simulink model "CCMDcMotor"  (R2022b)
settings: Solver=FixedStepDiscrete  StartTime=0.0  StopTime=10.0  FixedStep=0.01  RelTol=1e-3
model workspace (MAT data in the model file):
  NvMBlockDcMtrCalData : Simulink.Signal (value not decoded)
  NvMBlockDcMtrCalDataInit : Simulink.Parameter (value not decoded)
symbols that are objects in the model workspace (value not decoded): NvMBlockDcMtrCalDataInit
undefined symbols (not defined in the file or in its folder): DTCState, NvMBlockId, P_Std_ReturnType, Tle91ChlType, Tle91CmdType
(part 2 of 3)

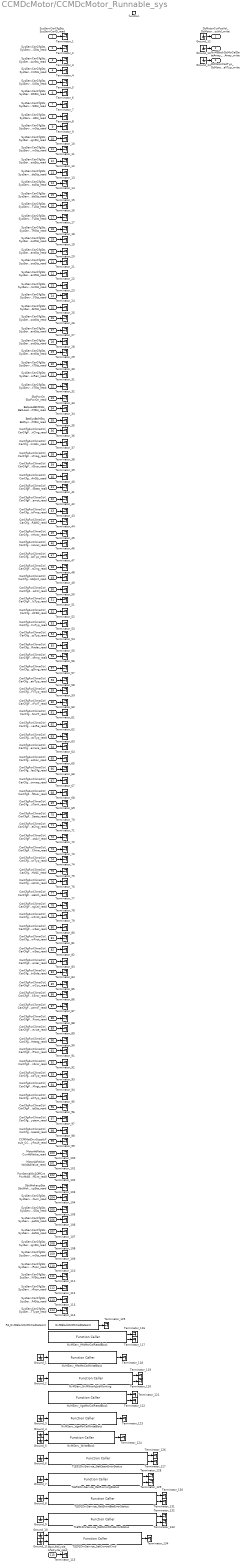
== system CCMDcMotor/CCMDcMotor_Runnable_sys ==
  SysServCarCfgSts_SysServCarID_read : Inport
  SysServCarCfgSts_SysServCCPAQSSts_read : Inport  Port=2
  SysServCarCfgSts_SysServCCPHEXSensorSts_read : Inport  Port=3
  SysServCarCfgSts_SysServCCPLin2AFUSts_read : Inport  Port=4
  SysServCarCfgSts_SysServCCPLin2AIUSts_read : Inport  Port=5
  SysServCarCfgSts_SysServCCPLin2CDSSts_read : Inport  Port=6
  SysServCarCfgSts_SysServCCPLin2PMSISts_read : Inport  Port=7
  SysServCarCfgSts_SysServCCPLin3VGASts_read : Inport  Port=8
  SysServCarCfgSts_SysServDUAirVentSnrSts_read : Inport  Port=9
  SysServCarCfgSts_SysServDUBlowerPwmSignSts_read : Inport  Port=10
  SysServCarCfgSts_SysServDUBlowerPwmSts_read : Inport  Port=11
  SysServCarCfgSts_SysServDUDCDefrostSts_read : Inport  Port=12
  SysServCarCfgSts_SysServDUDCModeSts_read : Inport  Port=13
  SysServCarCfgSts_SysServDUDCRecSts_read : Inport  Port=14
  SysServCarCfgSts_SysServDUDCSModeSts_read : Inport  Port=15
  SysServCarCfgSts_SysServDUDCSTLSts_read : Inport  Port=16
  SysServCarCfgSts_SysServDUDCTLSts_read : Inport  Port=17
  SysServCarCfgSts_SysServDUDCTRSts_read : Inport  Port=18
  SysServCarCfgSts_SysServDUFLFootSts_read : Inport  Port=19
  SysServCarCfgSts_SysServDUFLVentSts_read : Inport  Port=20
  SysServCarCfgSts_SysServDUFRFootSts_read : Inport  Port=21
  SysServCarCfgSts_SysServDUFRVentSts_read : Inport  Port=22
  SysServCarCfgSts_SysServDUIncarMotorSts_read : Inport  Port=23
  SysServCarCfgSts_SysServDULin1HBMFSts_read : Inport  Port=24
  SysServCarCfgSts_SysServDULin4HFAFSts_read : Inport  Port=25
  SysServCarCfgSts_SysServDUSFLFootSts_read : Inport  Port=26
  SysServCarCfgSts_SysServDUSFLVentSts_read : Inport  Port=27
  SysServCarCfgSts_SysServDUSFRFootSts_read : Inport  Port=28
  SysServCarCfgSts_SysServDUSFRVentSts_read : Inport  Port=29
  SysServCarCfgSts_SysServDUSnsrForFCmptmtTSts_read : Inport  Port=30
  SysServCarCfgSts_SysServDUSnsrForRair_read : Inport  Port=31
  SysServCarCfgSts_SysServDUSnsrForRCmptmtTSts_read : Inport  Port=32
  SbcPwrOn_SbcPwrOn_read : Inport  Port=33
  BatLoadBtrMSts_BatLoadBtrMSts_read : Inport  Port=34
  BatSysBtrMSts_BatSysBtrMSts_read : Inport  Port=35
  CarCfgForClimaCtrl_CarCfgForAirClng_read : Inport  Port=36
  CarCfgForClimaCtrl_CarCfgForAirClnrAdv_read : Inport  Port=37
  CarCfgForClimaCtrl_CarCfgForAirFrag_read : Inport  Port=38
  CarCfgForClimaCtrl_CarCfgForAirMtrlSnsr_read : Inport  Port=39
  CarCfgForClimaCtrl_CarCfgForAirQly_read : Inport  Port=40
  CarCfgForClimaCtrl_CarCfgForAmntOfSeat_read : Inport  Port=41
  CarCfgForClimaCtrl_CarCfgForAutDemst_read : Inport  Port=42
  CarCfgForClimaCtrl_CarCfgForAuxWtrPmp_read : Inport  Port=43
  CarCfgForClimaCtrl_CarCfgForBRAND_read : Inport  Port=44
  CarCfgForClimaCtrl_CarCfgForBypVlvInHvac_read : Inport  Port=45
  CarCfgForClimaCtrl_CarCfgForClsVlvInHvac_read : Inport  Port=46
  CarCfgForClimaCtrl_CarCfgForCmprAcTyp_read : Inport  Port=47
  CarCfgForClimaCtrl_CarCfgForCmptmtClng_read : Inport  Port=48
  CarCfgForClimaCtrl_CarCfgForElecVentAdjmt_read : Inport  Port=49
  CarCfgForClimaCtrl_CarCfgForExtVentnClimaCtrl_read : Inport  Port=50
  CarCfgForClimaCtrl_CarCfgForFlcTyp_read : Inport  Port=51
  CarCfgForClimaCtrl_CarCfgForFoldCSD_read : Inport  Port=52
  CarCfgForClimaCtrl_CarCfgForFuTyp_read : Inport  Port=53
  CarCfgForClimaCtrl_CarCfgForGlassTyp_read : Inport  Port=54
  CarCfgForClimaCtrl_CarCfgForHeadRadar_read : Inport  Port=55
  CarCfgForClimaCtrl_CarCfgForHeatPmp_read : Inport  Port=56
  CarCfgForClimaCtrl_CarCfgForHeatrDurgDrvg_read : Inport  Port=57
  CarCfgForClimaCtrl_CarCfgForHeatrTyp_read : Inport  Port=58
  CarCfgForClimaCtrl_CarCfgForHvacFilTyp_read : Inport  Port=59
  CarCfgForClimaCtrl_CarCfgForHvacFlrSnsrForT_read : Inport  Port=60
  CarCfgForClimaCtrl_CarCfgForHvacHeatrSnsrT_read : Inport  Port=61
  CarCfgForClimaCtrl_CarCfgForHvacRe_read : Inport  Port=62
  CarCfgForClimaCtrl_CarCfgForHvacTyp_read : Inport  Port=63
  CarCfgForClimaCtrl_CarCfgForInteriorViewCamera_read : Inport  Port=64
  CarCfgForClimaCtrl_CarCfgForLifeDetection_read : Inport  Port=65
  CarCfgForClimaCtrl_CarCfgForLoVoltElecCfg_read : Inport  Port=66
  CarCfgForClimaCtrl_CarCfgForNavSysandIntnmap_read : Inport  Port=67
  CarCfgForClimaCtrl_CarCfgForNrOfDoor_read : Inport  Port=68
  CarCfgForClimaCtrl_CarCfgForPipeInFuTank_read : Inport  Port=69
  CarCfgForClimaCtrl_CarCfgForPowerSeats_read : Inport  Port=70
  CarCfgForClimaCtrl_CarCfgForPreClng_read : Inport  Port=71
  CarCfgForClimaCtrl_CarCfgForPrkgCDSNotAvl_read : Inport  Port=72
  CarCfgForClimaCtrl_CarCfgForPrkgClima_read : Inport  Port=73
  CarCfgForClimaCtrl_CarCfgForPrpsnTyp_read : Inport  Port=74
  CarCfgForClimaCtrl_CarCfgForPTCInFrontHVAC_read : Inport  Port=75
  CarCfgForClimaCtrl_CarCfgForRemEngStrtPreCdn_read : Inport  Port=76
  CarCfgForClimaCtrl_CarCfgForRoofHatch_read : Inport  Port=77
  CarCfgForClimaCtrl_CarCfgForSeatHeatgCal_read : Inport  Port=78
  CarCfgForClimaCtrl_CarCfgForSeatHeatgForRowFirst_read : Inport  Port=79
  CarCfgForClimaCtrl_CarCfgForSeatHeatgForRowSec_read : Inport  Port=80
  CarCfgForClimaCtrl_CarCfgForSeatVentnForRowFirst_read : Inport  Port=81
  CarCfgForClimaCtrl_CarCfgForSeatVentnForRowSec_read : Inport  Port=82
  CarCfgForClimaCtrl_CarCfgForSecRowVentnCenter_read : Inport  Port=83
  CarCfgForClimaCtrl_CarCfgForSecRowVentnSide_read : Inport  Port=84
  CarCfgForClimaCtrl_CarCfgForSmallFlowCyc_read : Inport  Port=85
  CarCfgForClimaCtrl_CarCfgForSmkSnsr_read : Inport  Port=86
  CarCfgForClimaCtrl_CarCfgForSnsrForCmptmtT_read : Inport  Port=87
  CarCfgForClimaCtrl_CarCfgForSnsrHumPrsnt_read : Inport  Port=88
  CarCfgForClimaCtrl_CarCfgForStartDevice_read : Inport  Port=89
  CarCfgForClimaCtrl_CarCfgForSteerWhlHeatg_read : Inport  Port=90
  CarCfgForClimaCtrl_CarCfgForSteerWhlPosn_read : Inport  Port=91
  CarCfgForClimaCtrl_CarCfgForSunSnsr_read : Inport  Port=92
  CarCfgForClimaCtrl_CarCfgForTelmNodTyp_read : Inport  Port=93
  CarCfgForClimaCtrl_CarCfgForThermMngt_read : Inport  Port=94
  CarCfgForClimaCtrl_CarCfgForVehTyp_read : Inport  Port=95
  CarCfgForClimaCtrl_CarCfgForVgaMotorExistSts_read : Inport  Port=96
  CarCfgForClimaCtrl_CarCfgForWadingAssistSystem_read : Inport  Port=97
  CarCfgForClimaCtrl_CarCfgForWindHeatd_read : Inport  Port=98
  CCMMotDrvrSupplyFault_CCMMotDrvrSupplyFault_read : Inport  Port=99
  MotorAdValue_CurrAdValue_read : Inport  Port=100
  MotorAdValue_ValidAdValue_read : Inport  Port=101
  PwrGernalOn2OffCnt_PwrMdGernalOn2OffCnt_read : Inport  Port=102
  SbcWakeupSta_SbcWakeupSta_read : Inport  Port=103
  SysServCarCfgSts_SysServCCPBGMNum_read : Inport  Port=104
  SysServCarCfgSts_SysServCCPLin2AQSSts_read : Inport  Port=105
  SysServCarCfgSts_SysServDUBlowerPwmSignTypeSts_read : Inport  Port=106
  SysServCarCfgSts_SysServDUDCFLMdeSts_read : Inport  Port=107
  SysServCarCfgSts_SysServDUReBlowerPwmSignSts_read : Inport  Port=108
  SysServCarCfgSts_SysServDUReBlowerPwmSts_read : Inport  Port=109
  SysServCarCfgSts_SysServFrntHEXSnsrPosn_read : Inport  Port=110
  SysServCarCfgSts_SysServLowSideRETXVSts_read : Inport  Port=111
  SysServCarCfgSts_SysServReHEXSnsrPosn_read : Inport  Port=112
  SysServCarCfgSts_SysServRHFASts_read : Inport  Port=113
  SysServCarCfgSts_SysServSnsrForFCmptmtTType_read : Inport  Port=114
  BswLifeCycle_LifeCycle_read : Inport  Port=115
  function : TriggerPort  TriggerType=function-call  VariantControl=(inherit)  SampleTimeType=periodic  SampleTime=-1
  Ground_{1..14} (14 alike) : Ground
  NvMServ_HfaMtrCalReadBlock : FunctionCaller  FunctionPrototype=[Data,ERR] = NvMServ_HfaMtrCalReadBlock()  OutputArgumentSpecifications=uint8([1]), P_Std_ReturnType
  NvMServ_HfaMtrCalWriteBlock : FunctionCaller  FunctionPrototype=ERR = NvMServ_HfaMtrCalWriteBlock(Data)  InputArgumentSpecifications=uint8([1])  OutputArgumentSpecifications=P_Std_ReturnType
  NvMServ_NvMNotifyJobWorking : FunctionCaller  FunctionPrototype=[Status,ERR] = NvMServ_NvMNotifyJobWorking(BlockId)  InputArgumentSpecifications=NvMBlockId(0)  OutputArgumentSpecifications=uint8(1), P_Std_ReturnType
  NvMServ_VgaMtrCalReadBlock : FunctionCaller  FunctionPrototype=[Data,ERR] = NvMServ_VgaMtrCalReadBlock()  OutputArgumentSpecifications=uint8([1]), P_Std_ReturnType
  NvMServ_VgaMtrCalWriteBlock : FunctionCaller  FunctionPrototype=ERR = NvMServ_VgaMtrCalWriteBlock(Data)  InputArgumentSpecifications=uint8([1])  OutputArgumentSpecifications=P_Std_ReturnType
  NvMServ_WriteBlock : FunctionCaller  FunctionPrototype=ERR = NvMServ_WriteBlock(BlockId,Data)  InputArgumentSpecifications=NvMBlockId(0), uint8([1])  OutputArgumentSpecifications=P_Std_ReturnType
  PA_NvMBlockDcMtrCalDataInit : Constant  Value=NvMBlockDcMtrCalDataInit
  TLE91DrvService_GetOpenErrorStatus : FunctionCaller  FunctionPrototype=[Status,ERR] = TLE91DrvService_GetOpenErrorStatus(Channel)  InputArgumentSpecifications=Tle91ChlType(0)  OutputArgumentSpecifications=DTCState(0), P_Std_ReturnType
  TLE91DrvService_GetRunningStatus : FunctionCaller  FunctionPrototype=[Status,ERR] = TLE91DrvService_GetRunningStatus(Channel)  InputArgumentSpecifications=Tle91ChlType(0)  OutputArgumentSpecifications=DTCState(0), P_Std_ReturnType
  TLE91DrvService_GetShortBatErrorStatus : FunctionCaller  FunctionPrototype=[Status,ERR] = TLE91DrvService_GetShortBatErrorStatus(Channel)  InputArgumentSpecifications=Tle91ChlType(0)  OutputArgumentSpecifications=DTCState(0), P_Std_ReturnType
  TLE91DrvService_GetShortGndErrorStatus : FunctionCaller  FunctionPrototype=[Status,ERR] = TLE91DrvService_GetShortGndErrorStatus(Channel)  InputArgumentSpecifications=Tle91ChlType(0)  OutputArgumentSpecifications=DTCState(0), P_Std_ReturnType
  TLE91DrvService_SetControlCmd : FunctionCaller  FunctionPrototype=ERR = TLE91DrvService_SetControlCmd(Channel,DrvCmd)  InputArgumentSpecifications=Tle91ChlType(0), Tle91CmdType(0)  OutputArgumentSpecifications=P_Std_ReturnType
  Terminator_{1..134} (134 alike) : Terminator
  DcMotorCurPosVol_DcMotorCurPosVol_write : Outport
      settings: EnsureOutportIsVirtual=on
  NvMBlockDcMtrCalDataArray_NvMBlockDcMtrCalDataArray_write : Outport  Port=2
      settings: EnsureOutportIsVirtual=on
  DcMotorCaliTyp_DcMotorCaliTyp_write : Outport  Port=3
      settings: EnsureOutportIsVirtual=on
  wires:
  SysServCarCfgSts_SysServCarID_read/1 -> Terminator_1/1
  SysServCarCfgSts_SysServCCPAQSSts_read/1 -> Terminator_2/1
  SysServCarCfgSts_SysServCCPHEXSensorSts_read/1 -> Terminator_3/1
  SysServCarCfgSts_SysServCCPLin2AFUSts_read/1 -> Terminator_4/1
  SysServCarCfgSts_SysServCCPLin2AIUSts_read/1 -> Terminator_5/1
  SysServCarCfgSts_SysServCCPLin2CDSSts_read/1 -> Terminator_6/1
  SysServCarCfgSts_SysServCCPLin2PMSISts_read/1 -> Terminator_7/1
  SysServCarCfgSts_SysServCCPLin3VGASts_read/1 -> Terminator_8/1
  SysServCarCfgSts_SysServDUAirVentSnrSts_read/1 -> Terminator_9/1
  SysServCarCfgSts_SysServDUBlowerPwmSignSts_read/1 -> Terminator_10/1
  SysServCarCfgSts_SysServDUBlowerPwmSts_read/1 -> Terminator_11/1
  SysServCarCfgSts_SysServDUDCDefrostSts_read/1 -> Terminator_12/1
  SysServCarCfgSts_SysServDUDCModeSts_read/1 -> Terminator_13/1
  SysServCarCfgSts_SysServDUDCRecSts_read/1 -> Terminator_14/1
  SysServCarCfgSts_SysServDUDCSModeSts_read/1 -> Terminator_15/1
  SysServCarCfgSts_SysServDUDCSTLSts_read/1 -> Terminator_16/1
  SysServCarCfgSts_SysServDUDCTLSts_read/1 -> Terminator_17/1
  SysServCarCfgSts_SysServDUDCTRSts_read/1 -> Terminator_18/1
  SysServCarCfgSts_SysServDUFLFootSts_read/1 -> Terminator_19/1
  SysServCarCfgSts_SysServDUFLVentSts_read/1 -> Terminator_20/1
  SysServCarCfgSts_SysServDUFRFootSts_read/1 -> Terminator_21/1
  SysServCarCfgSts_SysServDUFRVentSts_read/1 -> Terminator_22/1
  SysServCarCfgSts_SysServDUIncarMotorSts_read/1 -> Terminator_23/1
  SysServCarCfgSts_SysServDULin1HBMFSts_read/1 -> Terminator_24/1
  SysServCarCfgSts_SysServDULin4HFAFSts_read/1 -> Terminator_25/1
  SysServCarCfgSts_SysServDUSFLFootSts_read/1 -> Terminator_26/1
  SysServCarCfgSts_SysServDUSFLVentSts_read/1 -> Terminator_27/1
  SysServCarCfgSts_SysServDUSFRFootSts_read/1 -> Terminator_28/1
  SysServCarCfgSts_SysServDUSFRVentSts_read/1 -> Terminator_29/1
  SysServCarCfgSts_SysServDUSnsrForFCmptmtTSts_read/1 -> Terminator_30/1
  SysServCarCfgSts_SysServDUSnsrForRair_read/1 -> Terminator_31/1
  SysServCarCfgSts_SysServDUSnsrForRCmptmtTSts_read/1 -> Terminator_32/1
  SbcPwrOn_SbcPwrOn_read/1 -> Terminator_33/1
  BatLoadBtrMSts_BatLoadBtrMSts_read/1 -> Terminator_34/1
  BatSysBtrMSts_BatSysBtrMSts_read/1 -> Terminator_35/1
  CarCfgForClimaCtrl_CarCfgForAirClng_read/1 -> Terminator_36/1
  CarCfgForClimaCtrl_CarCfgForAirClnrAdv_read/1 -> Terminator_37/1
  CarCfgForClimaCtrl_CarCfgForAirFrag_read/1 -> Terminator_38/1
  CarCfgForClimaCtrl_CarCfgForAirMtrlSnsr_read/1 -> Terminator_39/1
  CarCfgForClimaCtrl_CarCfgForAirQly_read/1 -> Terminator_40/1
  CarCfgForClimaCtrl_CarCfgForAmntOfSeat_read/1 -> Terminator_41/1
  CarCfgForClimaCtrl_CarCfgForAutDemst_read/1 -> Terminator_42/1
  CarCfgForClimaCtrl_CarCfgForAuxWtrPmp_read/1 -> Terminator_43/1
  CarCfgForClimaCtrl_CarCfgForBRAND_read/1 -> Terminator_44/1
  CarCfgForClimaCtrl_CarCfgForBypVlvInHvac_read/1 -> Terminator_45/1
  CarCfgForClimaCtrl_CarCfgForClsVlvInHvac_read/1 -> Terminator_46/1
  CarCfgForClimaCtrl_CarCfgForCmprAcTyp_read/1 -> Terminator_47/1
  CarCfgForClimaCtrl_CarCfgForCmptmtClng_read/1 -> Terminator_48/1
  CarCfgForClimaCtrl_CarCfgForElecVentAdjmt_read/1 -> Terminator_49/1
  CarCfgForClimaCtrl_CarCfgForExtVentnClimaCtrl_read/1 -> Terminator_50/1
  CarCfgForClimaCtrl_CarCfgForFlcTyp_read/1 -> Terminator_51/1
  CarCfgForClimaCtrl_CarCfgForFoldCSD_read/1 -> Terminator_52/1
  CarCfgForClimaCtrl_CarCfgForFuTyp_read/1 -> Terminator_53/1
  CarCfgForClimaCtrl_CarCfgForGlassTyp_read/1 -> Terminator_54/1
  CarCfgForClimaCtrl_CarCfgForHeadRadar_read/1 -> Terminator_55/1
  CarCfgForClimaCtrl_CarCfgForHeatPmp_read/1 -> Terminator_56/1
  CarCfgForClimaCtrl_CarCfgForHeatrDurgDrvg_read/1 -> Terminator_57/1
  CarCfgForClimaCtrl_CarCfgForHeatrTyp_read/1 -> Terminator_58/1
  CarCfgForClimaCtrl_CarCfgForHvacFilTyp_read/1 -> Terminator_59/1
  CarCfgForClimaCtrl_CarCfgForHvacFlrSnsrForT_read/1 -> Terminator_60/1
  CarCfgForClimaCtrl_CarCfgForHvacHeatrSnsrT_read/1 -> Terminator_61/1
  CarCfgForClimaCtrl_CarCfgForHvacRe_read/1 -> Terminator_62/1
  CarCfgForClimaCtrl_CarCfgForHvacTyp_read/1 -> Terminator_63/1
  CarCfgForClimaCtrl_CarCfgForInteriorViewCamera_read/1 -> Terminator_64/1
  CarCfgForClimaCtrl_CarCfgForLifeDetection_read/1 -> Terminator_65/1
  CarCfgForClimaCtrl_CarCfgForLoVoltElecCfg_read/1 -> Terminator_66/1
  CarCfgForClimaCtrl_CarCfgForNavSysandIntnmap_read/1 -> Terminator_67/1
  CarCfgForClimaCtrl_CarCfgForNrOfDoor_read/1 -> Terminator_68/1
  CarCfgForClimaCtrl_CarCfgForPipeInFuTank_read/1 -> Terminator_69/1
  CarCfgForClimaCtrl_CarCfgForPowerSeats_read/1 -> Terminator_70/1
  CarCfgForClimaCtrl_CarCfgForPreClng_read/1 -> Terminator_71/1
  CarCfgForClimaCtrl_CarCfgForPrkgCDSNotAvl_read/1 -> Terminator_72/1
  CarCfgForClimaCtrl_CarCfgForPrkgClima_read/1 -> Terminator_73/1
  CarCfgForClimaCtrl_CarCfgForPrpsnTyp_read/1 -> Terminator_74/1
  CarCfgForClimaCtrl_CarCfgForPTCInFrontHVAC_read/1 -> Terminator_75/1
  CarCfgForClimaCtrl_CarCfgForRemEngStrtPreCdn_read/1 -> Terminator_76/1
  CarCfgForClimaCtrl_CarCfgForRoofHatch_read/1 -> Terminator_77/1
  CarCfgForClimaCtrl_CarCfgForSeatHeatgCal_read/1 -> Terminator_78/1
  CarCfgForClimaCtrl_CarCfgForSeatHeatgForRowFirst_read/1 -> Terminator_79/1
  CarCfgForClimaCtrl_CarCfgForSeatHeatgForRowSec_read/1 -> Terminator_80/1
  CarCfgForClimaCtrl_CarCfgForSeatVentnForRowFirst_read/1 -> Terminator_81/1
  CarCfgForClimaCtrl_CarCfgForSeatVentnForRowSec_read/1 -> Terminator_82/1
  CarCfgForClimaCtrl_CarCfgForSecRowVentnCenter_read/1 -> Terminator_83/1
  CarCfgForClimaCtrl_CarCfgForSecRowVentnSide_read/1 -> Terminator_84/1
  CarCfgForClimaCtrl_CarCfgForSmallFlowCyc_read/1 -> Terminator_85/1
  CarCfgForClimaCtrl_CarCfgForSmkSnsr_read/1 -> Terminator_86/1
  CarCfgForClimaCtrl_CarCfgForSnsrForCmptmtT_read/1 -> Terminator_87/1
  CarCfgForClimaCtrl_CarCfgForSnsrHumPrsnt_read/1 -> Terminator_88/1
  CarCfgForClimaCtrl_CarCfgForStartDevice_read/1 -> Terminator_89/1
  CarCfgForClimaCtrl_CarCfgForSteerWhlHeatg_read/1 -> Terminator_90/1
  CarCfgForClimaCtrl_CarCfgForSteerWhlPosn_read/1 -> Terminator_91/1
  CarCfgForClimaCtrl_CarCfgForSunSnsr_read/1 -> Terminator_92/1
  CarCfgForClimaCtrl_CarCfgForTelmNodTyp_read/1 -> Terminator_93/1
  CarCfgForClimaCtrl_CarCfgForThermMngt_read/1 -> Terminator_94/1
  CarCfgForClimaCtrl_CarCfgForVehTyp_read/1 -> Terminator_95/1
  CarCfgForClimaCtrl_CarCfgForVgaMotorExistSts_read/1 -> Terminator_96/1
  CarCfgForClimaCtrl_CarCfgForWadingAssistSystem_read/1 -> Terminator_97/1
  CarCfgForClimaCtrl_CarCfgForWindHeatd_read/1 -> Terminator_98/1
  CCMMotDrvrSupplyFault_CCMMotDrvrSupplyFault_read/1 -> Terminator_99/1
  MotorAdValue_CurrAdValue_read/1 -> Terminator_100/1
  MotorAdValue_ValidAdValue_read/1 -> Terminator_101/1
  PwrGernalOn2OffCnt_PwrMdGernalOn2OffCnt_read/1 -> Terminator_102/1
  SbcWakeupSta_SbcWakeupSta_read/1 -> Terminator_103/1
  SysServCarCfgSts_SysServCCPBGMNum_read/1 -> Terminator_104/1
  SysServCarCfgSts_SysServCCPLin2AQSSts_read/1 -> Terminator_105/1
  SysServCarCfgSts_SysServDUBlowerPwmSignTypeSts_read/1 -> Terminator_106/1
  SysServCarCfgSts_SysServDUDCFLMdeSts_read/1 -> Terminator_107/1
  SysServCarCfgSts_SysServDUReBlowerPwmSignSts_read/1 -> Terminator_108/1
  SysServCarCfgSts_SysServDUReBlowerPwmSts_read/1 -> Terminator_109/1
  SysServCarCfgSts_SysServFrntHEXSnsrPosn_read/1 -> Terminator_110/1
  SysServCarCfgSts_SysServLowSideRETXVSts_read/1 -> Terminator_111/1
  SysServCarCfgSts_SysServReHEXSnsrPosn_read/1 -> Terminator_112/1
  SysServCarCfgSts_SysServRHFASts_read/1 -> Terminator_113/1
  SysServCarCfgSts_SysServSnsrForFCmptmtTType_read/1 -> Terminator_114/1
  BswLifeCycle_LifeCycle_read/1 -> Terminator_115/1
  NvMServ_HfaMtrCalReadBlock/1 -> Terminator_116/1
  NvMServ_HfaMtrCalReadBlock/2 -> Terminator_117/1
  Ground_1/1 -> NvMServ_HfaMtrCalWriteBlock/1
  NvMServ_HfaMtrCalWriteBlock/1 -> Terminator_118/1
  Ground_2/1 -> NvMServ_NvMNotifyJobWorking/1
  NvMServ_NvMNotifyJobWorking/1 -> Terminator_119/1
  NvMServ_NvMNotifyJobWorking/2 -> Terminator_120/1
  NvMServ_VgaMtrCalReadBlock/1 -> Terminator_121/1
  NvMServ_VgaMtrCalReadBlock/2 -> Terminator_122/1
  Ground_3/1 -> NvMServ_VgaMtrCalWriteBlock/1
  NvMServ_VgaMtrCalWriteBlock/1 -> Terminator_123/1
  Ground_4/1 -> NvMServ_WriteBlock/1
  Ground_5/1 -> NvMServ_WriteBlock/2
  NvMServ_WriteBlock/1 -> Terminator_124/1
  PA_NvMBlockDcMtrCalDataInit/1 -> Terminator_125/1
  Ground_6/1 -> TLE91DrvService_GetOpenErrorStatus/1
  TLE91DrvService_GetOpenErrorStatus/1 -> Terminator_126/1
  TLE91DrvService_GetOpenErrorStatus/2 -> Terminator_127/1
  Ground_7/1 -> TLE91DrvService_GetRunningStatus/1
  TLE91DrvService_GetRunningStatus/1 -> Terminator_128/1
  TLE91DrvService_GetRunningStatus/2 -> Terminator_129/1
  Ground_8/1 -> TLE91DrvService_GetShortBatErrorStatus/1
  TLE91DrvService_GetShortBatErrorStatus/1 -> Terminator_130/1
  TLE91DrvService_GetShortBatErrorStatus/2 -> Terminator_131/1
  Ground_9/1 -> TLE91DrvService_GetShortGndErrorStatus/1
  TLE91DrvService_GetShortGndErrorStatus/1 -> Terminator_132/1
  TLE91DrvService_GetShortGndErrorStatus/2 -> Terminator_133/1
  Ground_10/1 -> TLE91DrvService_SetControlCmd/1
  Ground_11/1 -> TLE91DrvService_SetControlCmd/2
  TLE91DrvService_SetControlCmd/1 -> Terminator_134/1
  Ground_12/1 -> DcMotorCurPosVol_DcMotorCurPosVol_write/1
  Ground_13/1 -> NvMBlockDcMtrCalDataArray_NvMBlockDcMtrCalDataArray_write/1
  Ground_14/1 -> DcMotorCaliTyp_DcMotorCaliTyp_write/1

== system CCMDcMotor/DcMotNvMClrService ==
  DcMotNvMClrService_ClearNvMData_sys : Subsystem

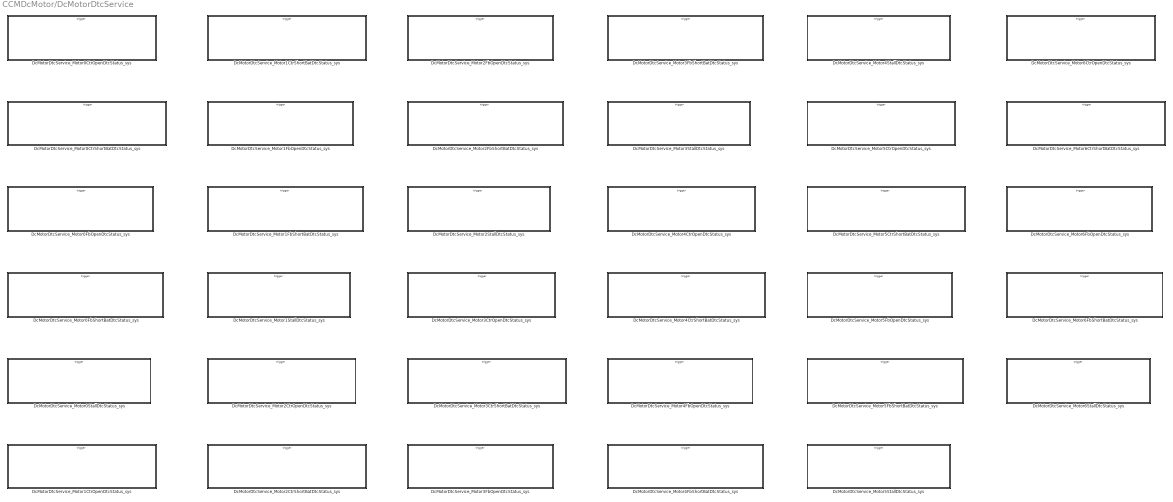
== system CCMDcMotor/DcMotorDtcService ==
  DcMotorDtcService_Motor0CtrOpenDtcStatus_sys : Subsystem
  DcMotorDtcService_Motor0CtrShortBatDtcStatus_sys : Subsystem
  DcMotorDtcService_Motor0FbOpenDtcStatus_sys : Subsystem
  DcMotorDtcService_Motor0FbShortBatDtcStatus_sys : Subsystem
  DcMotorDtcService_Motor0StallDtcStatus_sys : Subsystem
  DcMotorDtcService_Motor1CtrOpenDtcStatus_sys : Subsystem
  DcMotorDtcService_Motor1CtrShortBatDtcStatus_sys : Subsystem
  DcMotorDtcService_Motor1FbOpenDtcStatus_sys : Subsystem
  DcMotorDtcService_Motor1FbShortBatDtcStatus_sys : Subsystem
  DcMotorDtcService_Motor1StallDtcStatus_sys : Subsystem
  DcMotorDtcService_Motor2CtrOpenDtcStatus_sys : Subsystem
  DcMotorDtcService_Motor2CtrShortBatDtcStatus_sys : Subsystem
  DcMotorDtcService_Motor2FbOpenDtcStatus_sys : Subsystem
  DcMotorDtcService_Motor2FbShortBatDtcStatus_sys : Subsystem
  DcMotorDtcService_Motor2StallDtcStatus_sys : Subsystem
  DcMotorDtcService_Motor3CtrOpenDtcStatus_sys : Subsystem
  DcMotorDtcService_Motor3CtrShortBatDtcStatus_sys : Subsystem
  DcMotorDtcService_Motor3FbOpenDtcStatus_sys : Subsystem
  DcMotorDtcService_Motor3FbShortBatDtcStatus_sys : Subsystem
  DcMotorDtcService_Motor3StallDtcStatus_sys : Subsystem
  DcMotorDtcService_Motor4CtrOpenDtcStatus_sys : Subsystem
  DcMotorDtcService_Motor4CtrShortBatDtcStatus_sys : Subsystem
  DcMotorDtcService_Motor4FbOpenDtcStatus_sys : Subsystem
  DcMotorDtcService_Motor4FbShortBatDtcStatus_sys : Subsystem
  DcMotorDtcService_Motor4StallDtcStatus_sys : Subsystem
  DcMotorDtcService_Motor5CtrOpenDtcStatus_sys : Subsystem
  DcMotorDtcService_Motor5CtrShortBatDtcStatus_sys : Subsystem
  DcMotorDtcService_Motor5FbOpenDtcStatus_sys : Subsystem
  DcMotorDtcService_Motor5FbShortBatDtcStatus_sys : Subsystem
  DcMotorDtcService_Motor5StallDtcStatus_sys : Subsystem
  DcMotorDtcService_Motor6CtrOpenDtcStatus_sys : Subsystem
  DcMotorDtcService_Motor6CtrShortBatDtcStatus_sys : Subsystem
  DcMotorDtcService_Motor6FbOpenDtcStatus_sys : Subsystem
  DcMotorDtcService_Motor6FbShortBatDtcStatus_sys : Subsystem
  DcMotorDtcService_Motor6StallDtcStatus_sys : Subsystem

== system CCMDcMotor/DcMotorIOCtrlService ==
  DcMotorIOCtrlService_FreezeMotorCurrentPosition_sys : Subsystem
  DcMotorIOCtrlService_MotorRetCtlToECU_sys : Subsystem
  DcMotorIOCtrlService_MotorShortTermAdj_sys : Subsystem
